simulink model "MX_Gateway"  (Simulink 9.0)
settings: Solver=FixedStepDiscrete  StartTime=0.0  StopTime=100  FixedStep=0.01  RelTol=1e-3
values from neighbouring files (symbols this model uses):
  ACTIVE = Bobcat.Parameter; CreateDefine( ACTIVE                         ,1                          ,'Define' ,'defines.h' )    [defines.m]
  ACTIVE_MODE_ONE = Bobcat.Parameter; CreateDefine( ACTIVE_MODE_ONE                ,19                         ,'Define' ,'defines.h' )    [defines.m]
  AUTHENTICATED = Bobcat.Parameter; CreateDefine( AUTHENTICATED                  ,1                          ,'Define' ,'defines.h' )    [defines.m]
  AUX_EMEA_CONTROLS = Bobcat.Parameter; CreateDefine( AUX_EMEA_CONTROLS  ,1   ,'Define' ,'defines.h' )    [enums.m]
  AUX_FLOW_HIGH = Bobcat.Parameter; CreateDefine( AUX_FLOW_HIGH           ,4  ,'Define' ,'defines.h' )    [enums.m]
  AUX_FLOW_INACTIVE = Bobcat.Parameter; CreateDefine( AUX_FLOW_INACTIVE       ,0  ,'Define' ,'defines.h' )    [enums.m]
  AUX_FLOW_LOW = Bobcat.Parameter; CreateDefine( AUX_FLOW_LOW            ,1  ,'Define' ,'defines.h' )    [enums.m]
  AUX_FLOW_MEDIUM = Bobcat.Parameter; CreateDefine( AUX_FLOW_MEDIUM         ,2  ,'Define' ,'defines.h' )    [enums.m]
  AUX_FLOW_STANDARD = Bobcat.Parameter; CreateDefine( AUX_FLOW_STANDARD       ,3  ,'Define' ,'defines.h' )    [enums.m]
  AUX_NON_FUNCTIONAL = Bobcat.Parameter; CreateDefine( AUX_NON_FUNCTIONAL ,255 ,'Define' ,'defines.h' )    [enums.m]
  BASE_ACTIVE = Bobcat.Parameter; CreateDefine( BASE_ACTIVE                    ,253                        ,'Define' ,'defines.h' )    [defines.m]
  BASE_DETENT = Bobcat.Parameter; CreateDefine( BASE_DETENT                    ,1                          ,'Define' ,'defines.h' )    [defines.m]
  BEEP_NONE = Bobcat.Parameter; CreateDefine( BEEP_NONE                      ,255                        ,'Define' ,'defines.h' )    [defines.m]
  CD_AIR_FILTER_DEBOUNCE_NORMAL = Bobcat.Parameter; CreateAliasCD( CD_AIR_FILTER_DEBOUNCE_NORMAL                        ,uint16(500)                ,'ConstantDataPointer' ,'CONSTANT_DATA' ,'AIR_FILTER_DEBOUNCE_NORMAL')    [cdata.m]    (also in hc12_cdata.m; m4_cdata.m)
  CD_AIR_FILTER_DEBOUNCE_NOT_CONNECTED = Bobcat.Parameter; CreateAliasCD( CD_AIR_FILTER_DEBOUNCE_NOT_CONNECTED                 ,uint16(3000)               ,'ConstantDataPointer' ,'CONSTANT_DATA' ,'AIR_FILTER_DEBOUNCE_NOT_CONNECTED')    [cdata.m]    (also in hc12_cdata.m; m4_cdata.m)
  CD_AIR_FILTER_DEBOUNCE_PLUGGED = Bobcat.Parameter; CreateAliasCD( CD_AIR_FILTER_DEBOUNCE_PLUGGED                       ,uint16(3000)               ,'ConstantDataPointer' ,'CONSTANT_DATA' ,'AIR_FILTER_DEBOUNCE_PLUGGED')    [cdata.m]    (also in hc12_cdata.m; m4_cdata.m)
  CD_AIR_FILTER_FUNCTION = Bobcat.Parameter; CreateAliasCD( CD_AIR_FILTER_FUNCTION                               ,uint8(255)                 ,'ConstantDataPointer' ,'CONSTANT_DATA' ,'AIR_FILTER_FUNCTION')    [cdata.m]    (also in hc12_cdata.m; m4_cdata.m)
  CD_AIR_FILTER_PLUGGED_WEIGHT = Bobcat.Parameter; CreateAliasCD( CD_AIR_FILTER_PLUGGED_WEIGHT                         ,uint8(2)                   ,'ConstantDataPointer' ,'CONSTANT_DATA' ,'AIR_FILTER_PLUGGED_WEIGHT')    [cdata.m]    (also in hc12_cdata.m; m4_cdata.m)
  CD_AIR_FILTER_UNPLUGGED_WEIGHT = Bobcat.Parameter; CreateAliasCD( CD_AIR_FILTER_UNPLUGGED_WEIGHT                       ,uint8(1)                   ,'ConstantDataPointer' ,'CONSTANT_DATA' ,'AIR_FILTER_UNPLUGGED_WEIGHT')    [cdata.m]    (also in hc12_cdata.m; m4_cdata.m)
  CD_AUTOIDLE_ARMBAR_DELAY = Bobcat.Parameter; CreateAliasCD( CD_AUTOIDLE_ARMBAR_DELAY                             ,uint8(2)                   ,'ConstantDataPointer' ,'CONSTANT_DATA' ,'AUTOIDLE_ARMBAR_DELAY')    [cdata.m]    (also in hc12_cdata.m; m4_cdata.m)
  CD_AUTOIDLE_FUNCTION = Bobcat.Parameter; CreateAliasCD( CD_AUTOIDLE_FUNCTION                                 ,uint8(255)                 ,'ConstantDataPointer' ,'CONSTANT_DATA' ,'AUTOIDLE_FUNCTION')    [cdata.m]    (also in hc12_cdata.m; m4_cdata.m)
  CD_AUTOIDLE_IDLE_DELAY = Bobcat.Parameter; CreateAliasCD( CD_AUTOIDLE_IDLE_DELAY                               ,uint8(40)                  ,'ConstantDataPointer' ,'CONSTANT_DATA' ,'AUTOIDLE_IDLE_DELAY')    [cdata.m]    (also in hc12_cdata.m; m4_cdata.m)
  CD_AUTOIDLE_LOW_IDLE_START = Bobcat.Parameter; CreateAliasCD( CD_AUTOIDLE_LOW_IDLE_START                           ,uint8(255)                 ,'ConstantDataPointer' ,'CONSTANT_DATA' ,'AUTOIDLE_LOW_IDLE_START')    [cdata.m]    (also in hc12_cdata.m; m4_cdata.m)
  CD_AUTOIDLE_PRESSURE_OFF = Bobcat.Parameter; CreateAliasCD( CD_AUTOIDLE_PRESSURE_OFF                             ,uint16(300)                ,'ConstantDataPointer' ,'CONSTANT_DATA' ,'AUTOIDLE_PRESSURE_OFF')    [cdata.m]    (also in hc12_cdata.m; m4_cdata.m)
  CD_AUTOIDLE_PRESSURE_ON = Bobcat.Parameter; CreateAliasCD( CD_AUTOIDLE_PRESSURE_ON                              ,uint16(270)                ,'ConstantDataPointer' ,'CONSTANT_DATA' ,'AUTOIDLE_PRESSURE_ON')    [cdata.m]    (also in hc12_cdata.m; m4_cdata.m)
  CD_AUTOSHIFT_FUNCTION = Bobcat.Parameter; CreateAliasCD( CD_AUTOSHIFT_FUNCTION                                ,uint8(255)                 ,'ConstantDataPointer' ,'CONSTANT_DATA' ,'AUTOSHIFT_FUNCTION')    [cdata.m]    (also in hc12_cdata.m; m4_cdata.m)
  CD_AUTOSHIFT_PHIGH1 = Bobcat.Parameter; CreateAliasCD( CD_AUTOSHIFT_PHIGH1                                  ,uint16(205)                ,'ConstantDataPointer' ,'CONSTANT_DATA' ,'AUTOSHIFT_PHIGH1')    [cdata.m]    (also in hc12_cdata.m; m4_cdata.m)
  CD_AUTOSHIFT_PHIGH2 = Bobcat.Parameter; CreateAliasCD( CD_AUTOSHIFT_PHIGH2                                  ,uint16(193)                ,'ConstantDataPointer' ,'CONSTANT_DATA' ,'AUTOSHIFT_PHIGH2')    [cdata.m]    (also in hc12_cdata.m; m4_cdata.m)
  CD_AUTOSHIFT_PHIGH_TEMP1 = Bobcat.Parameter; CreateAliasCD( CD_AUTOSHIFT_PHIGH_TEMP1                             ,int16(70)                  ,'ConstantDataPointer' ,'CONSTANT_DATA' ,'AUTOSHIFT_PHIGH_TEMP1')    [cdata.m]    (also in hc12_cdata.m; m4_cdata.m)
  CD_AUTOSHIFT_PHIGH_TEMP2 = Bobcat.Parameter; CreateAliasCD( CD_AUTOSHIFT_PHIGH_TEMP2                             ,int16(160)                 ,'ConstantDataPointer' ,'CONSTANT_DATA' ,'AUTOSHIFT_PHIGH_TEMP2')    [cdata.m]    (also in hc12_cdata.m; m4_cdata.m)
  CD_AUTOSHIFT_PLOW1 = Bobcat.Parameter; CreateAliasCD( CD_AUTOSHIFT_PLOW1                                   ,uint16(130)                ,'ConstantDataPointer' ,'CONSTANT_DATA' ,'AUTOSHIFT_PLOW1')    [cdata.m]    (also in hc12_cdata.m; m4_cdata.m)
  CD_AUTOSHIFT_PLOW2 = Bobcat.Parameter; CreateAliasCD( CD_AUTOSHIFT_PLOW2                                   ,uint16(100)                ,'ConstantDataPointer' ,'CONSTANT_DATA' ,'AUTOSHIFT_PLOW2')    [cdata.m]    (also in hc12_cdata.m; m4_cdata.m)
  CD_AUTOSHIFT_PLOW_TEMP1 = Bobcat.Parameter; CreateAliasCD( CD_AUTOSHIFT_PLOW_TEMP1                              ,int16(20)                  ,'ConstantDataPointer' ,'CONSTANT_DATA' ,'AUTOSHIFT_PLOW_TEMP1')    [cdata.m]    (also in hc12_cdata.m; m4_cdata.m)
  CD_AUTOSHIFT_PLOW_TEMP2 = Bobcat.Parameter; CreateAliasCD( CD_AUTOSHIFT_PLOW_TEMP2                              ,int16(80)                  ,'ConstantDataPointer' ,'CONSTANT_DATA' ,'AUTOSHIFT_PLOW_TEMP2')    [cdata.m]    (also in hc12_cdata.m; m4_cdata.m)
  CD_AUTOSHIFT_TRAVEL_DELAY = Bobcat.Parameter; CreateAliasCD( CD_AUTOSHIFT_TRAVEL_DELAY                            ,uint8(32)                  ,'ConstantDataPointer' ,'CONSTANT_DATA' ,'AUTOSHIFT_TRAVEL_DELAY')    [cdata.m]    (also in hc12_cdata.m; m4_cdata.m)
  CD_AUX2_CRACKPOINT_CURRENT = Bobcat.Parameter; CreateAliasCD( CD_AUX2_CRACKPOINT_CURRENT                           ,fi(0,0,16,12,'hex','0c28') ,'ConstantDataPointer' ,'CONSTANT_DATA' ,'AUX2_CRACKPOINT_CURRENT')    [cdata.m]    (also in hc12_cdata.m; m4_cdata.m)
  CD_AUX2_FLOW_LOW_FUNCTION = Bobcat.Parameter; CreateAliasCD( CD_AUX2_FLOW_LOW_FUNCTION                            ,uint8(255)                 ,'ConstantDataPointer' ,'CONSTANT_DATA' ,'AUX2_FLOW_LOW_FUNCTION')    [cdata.m]    (also in hc12_cdata.m; m4_cdata.m)
  CD_AUX2_FLOW_MED_FUNCTION = Bobcat.Parameter; CreateAliasCD( CD_AUX2_FLOW_MED_FUNCTION                            ,uint8(255)                 ,'ConstantDataPointer' ,'CONSTANT_DATA' ,'AUX2_FLOW_MED_FUNCTION')    [cdata.m]    (also in hc12_cdata.m; m4_cdata.m)
  CD_AUX2_HI_FLOW_PERCENT = Bobcat.Parameter; CreateAliasCD( CD_AUX2_HI_FLOW_PERCENT                              ,fi(0,0,16,16,'hex','ffff') ,'ConstantDataPointer' ,'CONSTANT_DATA' ,'AUX2_HI_FLOW_PERCENT')    [cdata.m]    (also in hc12_cdata.m; m4_cdata.m)
  CD_AUX2_LOW_FLOW_PERCENT = Bobcat.Parameter; CreateAliasCD( CD_AUX2_LOW_FLOW_PERCENT                             ,fi(0,0,16,16,'hex','7333') ,'ConstantDataPointer' ,'CONSTANT_DATA' ,'AUX2_LOW_FLOW_PERCENT')    [cdata.m]    (also in hc12_cdata.m; m4_cdata.m)
  CD_AUX2_MAX_FLOW_CURRENT = Bobcat.Parameter; CreateAliasCD( CD_AUX2_MAX_FLOW_CURRENT                             ,fi(0,0,16,12,'hex','14cc') ,'ConstantDataPointer' ,'CONSTANT_DATA' ,'AUX2_MAX_FLOW_CURRENT')    [cdata.m]    (also in hc12_cdata.m; m4_cdata.m)
  CD_AUX2_MED_FLOW_PERCENT = Bobcat.Parameter; CreateAliasCD( CD_AUX2_MED_FLOW_PERCENT                             ,fi(0,0,16,16,'hex','9999') ,'ConstantDataPointer' ,'CONSTANT_DATA' ,'AUX2_MED_FLOW_PERCENT')    [cdata.m]    (also in hc12_cdata.m; m4_cdata.m)
  CD_AUX_CRACKPOINT_CURRENT = Bobcat.Parameter; CreateAliasCD( CD_AUX_CRACKPOINT_CURRENT                            ,fi(0,0,16,12,'hex','0000') ,'ConstantDataPointer' ,'CONSTANT_DATA' ,'AUX_CRACKPOINT_CURRENT')    [cdata.m]    (also in hc12_cdata.m; m4_cdata.m)
  CD_AUX_DETENT_FLASH_TIME = Bobcat.Parameter; CreateAliasCD( CD_AUX_DETENT_FLASH_TIME                             ,uint16(72)                 ,'ConstantDataPointer' ,'CONSTANT_DATA' ,'AUX_DETENT_FLASH_TIME')    [cdata.m]    (also in hc12_cdata.m; m4_cdata.m)
  CD_AUX_DETENT_HOLD_TIME = Bobcat.Parameter; CreateAliasCD( CD_AUX_DETENT_HOLD_TIME                              ,uint16(100)                ,'ConstantDataPointer' ,'CONSTANT_DATA' ,'AUX_DETENT_HOLD_TIME')    [cdata.m]    (also in hc12_cdata.m; m4_cdata.m)
  CD_AUX_FULL_ON_AUX1 = Bobcat.Parameter; CreateAliasCD( CD_AUX_FULL_ON_AUX1                                  ,fi(0,0,16,12,'hex','10cd') ,'ConstantDataPointer' ,'CONSTANT_DATA' ,'AUX_FULL_ON_AUX1')    [cdata.m]    (also in hc12_cdata.m; m4_cdata.m)
  CD_AUX_FULL_ON_AUX2 = Bobcat.Parameter; CreateAliasCD( CD_AUX_FULL_ON_AUX2                                  ,fi(0,0,16,12,'hex','139d') ,'ConstantDataPointer' ,'CONSTANT_DATA' ,'AUX_FULL_ON_AUX2')    [cdata.m]    (also in hc12_cdata.m; m4_cdata.m)
  CD_AUX_FULL_ON_AUX3 = Bobcat.Parameter; CreateAliasCD( CD_AUX_FULL_ON_AUX3                                  ,fi(0,0,16,12,'hex','17d7') ,'ConstantDataPointer' ,'CONSTANT_DATA' ,'AUX_FULL_ON_AUX3')    [cdata.m]    (also in hc12_cdata.m; m4_cdata.m)
  CD_AUX_FUNCTION = Bobcat.Parameter; CreateAliasCD( CD_AUX_FUNCTION                                      ,uint8(0)                   ,'ConstantDataPointer' ,'CONSTANT_DATA' ,'AUX_FUNCTION')    [cdata.m]    (also in hc12_cdata.m; m4_cdata.m)
  CD_AUX_RATE_LIMIT_CRACKPOINT = Bobcat.Parameter; CreateAliasCD( CD_AUX_RATE_LIMIT_CRACKPOINT                         ,fi(0,0,16,12,'hex','0800') ,'ConstantDataPointer' ,'CONSTANT_DATA' ,'AUX_RATE_LIMIT_CRACKPOINT')    [cdata.m]    (also in hc12_cdata.m; m4_cdata.m)
  CD_AUX_RATE_LIMIT_CRACKPOINT_RATE = Bobcat.Parameter; CreateAliasCD( CD_AUX_RATE_LIMIT_CRACKPOINT_RATE                    ,fi(0,1,32,12,'int',102400) ,'ConstantDataPointer' ,'CONSTANT_DATA' ,'AUX_RATE_LIMIT_CRACKPOINT_RATE')    [cdata.m]    (also in hc12_cdata.m; m4_cdata.m)
  CD_AUX_RATE_LIMIT_FUNCTION = Bobcat.Parameter; CreateAliasCD( CD_AUX_RATE_LIMIT_FUNCTION                           ,uint8(255)                 ,'ConstantDataPointer' ,'CONSTANT_DATA' ,'AUX_RATE_LIMIT_FUNCTION')    [cdata.m]    (also in hc12_cdata.m; m4_cdata.m)
  CD_AUX_RATE_LIMIT_MAX_ENG_SPD = Bobcat.Parameter; CreateAliasCD( CD_AUX_RATE_LIMIT_MAX_ENG_SPD                        ,uint16(1600)               ,'ConstantDataPointer' ,'CONSTANT_DATA' ,'AUX_RATE_LIMIT_MAX_ENG_SPD')    [cdata.m]    (also in hc12_cdata.m; m4_cdata.m)
  CD_AUX_RATE_LIMIT_MIN_ENG_SPD = Bobcat.Parameter; CreateAliasCD( CD_AUX_RATE_LIMIT_MIN_ENG_SPD                        ,uint16(100)                ,'ConstantDataPointer' ,'CONSTANT_DATA' ,'AUX_RATE_LIMIT_MIN_ENG_SPD')    [cdata.m]    (also in hc12_cdata.m; m4_cdata.m)
  CD_AUX_RATE_LIMIT_NORMAL_RATE = Bobcat.Parameter; CreateAliasCD( CD_AUX_RATE_LIMIT_NORMAL_RATE                        ,fi(0,1,32,12,'int',2048)   ,'ConstantDataPointer' ,'CONSTANT_DATA' ,'AUX_RATE_LIMIT_NORMAL_RATE')    [cdata.m]    (also in hc12_cdata.m; m4_cdata.m)
  CD_AUXPOT_CAL_DEADBAND_HIGH = Bobcat.Parameter; CreateAliasCD( CD_AUXPOT_CAL_DEADBAND_HIGH                          ,uint16(574)                ,'ConstantDataPointer' ,'CONSTANT_DATA' ,'AUXPOT_CAL_DEADBAND_HIGH')    [cdata.m]    (also in hc12_cdata.m; m4_cdata.m)
  CD_AUXPOT_CAL_DEADBAND_LOW = Bobcat.Parameter; CreateAliasCD( CD_AUXPOT_CAL_DEADBAND_LOW                           ,uint16(424)                ,'ConstantDataPointer' ,'CONSTANT_DATA' ,'AUXPOT_CAL_DEADBAND_LOW')    [cdata.m]    (also in hc12_cdata.m; m4_cdata.m)
  CD_AUXPOT_CENTER_RATIO = Bobcat.Parameter; CreateAliasCD( CD_AUXPOT_CENTER_RATIO                               ,uint16(500)                ,'ConstantDataPointer' ,'CONSTANT_DATA' ,'AUXPOT_CENTER_RATIO')    [cdata.m]    (also in hc12_cdata.m; m4_cdata.m)
  CD_AUXPOT_DEADBAND = Bobcat.Parameter; CreateAliasCD( CD_AUXPOT_DEADBAND                                   ,uint8(38)                  ,'ConstantDataPointer' ,'CONSTANT_DATA' ,'AUXPOT_DEADBAND')    [cdata.m]    (also in hc12_cdata.m; m4_cdata.m)
  CD_AUXPOT_FUNCTION = Bobcat.Parameter; CreateAliasCD( CD_AUXPOT_FUNCTION                                   ,uint8(0)                   ,'ConstantDataPointer' ,'CONSTANT_DATA' ,'AUXPOT_FUNCTION')    [cdata.m]    (also in hc12_cdata.m; m4_cdata.m)
  CD_AUXPOT_RATIO_GAIN = Bobcat.Parameter; CreateAliasCD( CD_AUXPOT_RATIO_GAIN                                 ,uint16(1000)               ,'ConstantDataPointer' ,'CONSTANT_DATA' ,'AUXPOT_RATIO_GAIN')    [cdata.m]    (also in hc12_cdata.m; m4_cdata.m)
  CD_CAN_THROTTLE_FUNCTION = Bobcat.Parameter; CreateAliasCD( CD_CAN_THROTTLE_FUNCTION                             ,uint8(255)                 ,'ConstantDataPointer' ,'CONSTANT_DATA' ,'CAN_THROTTLE_FUNCTION')    [cdata.m]    (also in hc12_cdata.m; m4_cdata.m)
  CD_CAN_THROTTLE_REVERSE = Bobcat.Parameter; CreateAliasCD( CD_CAN_THROTTLE_REVERSE                              ,uint8(255)                 ,'ConstantDataPointer' ,'CONSTANT_DATA' ,'CAN_THROTTLE_REVERSE')    [cdata.m]    (also in hc12_cdata.m; m4_cdata.m)
  CD_CONSOLE_DEBOUNCE_ABSENT = Bobcat.Parameter; CreateAliasCD( CD_CONSOLE_DEBOUNCE_ABSENT                           ,uint16(12)                 ,'ConstantDataPointer' ,'CONSTANT_DATA' ,'CONSOLE_DEBOUNCE_ABSENT')    [cdata.m]    (also in hc12_cdata.m; m4_cdata.m)
  CD_CONSOLE_DEBOUNCE_OORH = Bobcat.Parameter; CreateAliasCD( CD_CONSOLE_DEBOUNCE_OORH                             ,uint16(12)                 ,'ConstantDataPointer' ,'CONSTANT_DATA' ,'CONSOLE_DEBOUNCE_OORH')    [cdata.m]    (also in hc12_cdata.m; m4_cdata.m)
  CD_CONSOLE_DEBOUNCE_OORL = Bobcat.Parameter; CreateAliasCD( CD_CONSOLE_DEBOUNCE_OORL                             ,uint16(12)                 ,'ConstantDataPointer' ,'CONSTANT_DATA' ,'CONSOLE_DEBOUNCE_OORL')    [cdata.m]    (also in hc12_cdata.m; m4_cdata.m)
  CD_CONSOLE_DEBOUNCE_PRESENT = Bobcat.Parameter; CreateAliasCD( CD_CONSOLE_DEBOUNCE_PRESENT                          ,uint16(12)                 ,'ConstantDataPointer' ,'CONSTANT_DATA' ,'CONSOLE_DEBOUNCE_PRESENT')    [cdata.m]    (also in hc12_cdata.m; m4_cdata.m)
  CD_CONSOLE_FUNCTION = Bobcat.Parameter; CreateAliasCD( CD_CONSOLE_FUNCTION                                  ,uint8(0)                   ,'ConstantDataPointer' ,'CONSTANT_DATA' ,'CONSOLE_FUNCTION')    [cdata.m]    (also in hc12_cdata.m; m4_cdata.m)
  CD_CONSOLE_OORH = Bobcat.Parameter; CreateAliasCD( CD_CONSOLE_OORH                                      ,uint16(740)                ,'ConstantDataPointer' ,'CONSTANT_DATA' ,'CONSOLE_OORH')    [cdata.m]    (also in hc12_cdata.m; m4_cdata.m)
  CD_CONSOLE_OORL = Bobcat.Parameter; CreateAliasCD( CD_CONSOLE_OORL                                      ,uint16(10)                 ,'ConstantDataPointer' ,'CONSTANT_DATA' ,'CONSOLE_OORL')    [cdata.m]    (also in hc12_cdata.m; m4_cdata.m)
  CD_CONSOLE_PRESENT_HIGH = Bobcat.Parameter; CreateAliasCD( CD_CONSOLE_PRESENT_HIGH                              ,uint16(515)                ,'ConstantDataPointer' ,'CONSTANT_DATA' ,'CONSOLE_PRESENT_HIGH')    [cdata.m]    (also in hc12_cdata.m; m4_cdata.m)
  CD_CONSOLE_PRESENT_LOW = Bobcat.Parameter; CreateAliasCD( CD_CONSOLE_PRESENT_LOW                               ,uint16(375)                ,'ConstantDataPointer' ,'CONSTANT_DATA' ,'CONSOLE_PRESENT_LOW')    [cdata.m]    (also in hc12_cdata.m; m4_cdata.m)
  CD_DELUXE_GEN5_5_FUNCTION = Bobcat.Parameter; CreateAliasCD( CD_DELUXE_GEN5_5_FUNCTION                            ,uint8(255)                 ,'ConstantDataPointer' ,'CONSTANT_DATA' ,'DELUXE_GEN5_5_FUNCTION')    [cdata.m]    (also in hc12_cdata.m; m4_cdata.m)
  CD_DELUXE_GEN5_FUNCTION = Bobcat.Parameter; CreateAliasCD( CD_DELUXE_GEN5_FUNCTION                              ,uint8(255)                 ,'ConstantDataPointer' ,'CONSTANT_DATA' ,'DELUXE_GEN5_FUNCTION')    [cdata.m]    (also in hc12_cdata.m; m4_cdata.m)
  CD_DERATE_ENGINE_SPEED_DEBOUNCE_HIGH = Bobcat.Parameter; CreateAliasCD( CD_DERATE_ENGINE_SPEED_DEBOUNCE_HIGH                 ,uint16(200)                ,'ConstantDataPointer' ,'CONSTANT_DATA' ,'DERATE_ENGINE_SPEED_DEBOUNCE_HIGH')    [cdata.m]    (also in hc12_cdata.m; m4_cdata.m)
  CD_DERATE_ENGINE_SPEED_DEBOUNCE_SHUTDOWN = Bobcat.Parameter; CreateAliasCD( CD_DERATE_ENGINE_SPEED_DEBOUNCE_SHUTDOWN             ,uint16(1000)               ,'ConstantDataPointer' ,'CONSTANT_DATA' ,'DERATE_ENGINE_SPEED_DEBOUNCE_SHUTDOWN')    [cdata.m]    (also in hc12_cdata.m; m4_cdata.m)
  CD_DERATE_ENGINE_SPEED_HIGH_OFFSET = Bobcat.Parameter; CreateAliasCD( CD_DERATE_ENGINE_SPEED_HIGH_OFFSET                   ,uint16(200)                ,'ConstantDataPointer' ,'CONSTANT_DATA' ,'DERATE_ENGINE_SPEED_HIGH_OFFSET')    [cdata.m]    (also in hc12_cdata.m; m4_cdata.m)
  CD_DERATE_METHOD = Bobcat.Parameter; CreateAliasCD( CD_DERATE_METHOD                                     ,uint8(0)                   ,'ConstantDataPointer' ,'CONSTANT_DATA' ,'DERATE_METHOD')    [cdata.m]    (also in hc12_cdata.m; m4_cdata.m)
  CD_DUMP_VALVE_FUNCTION = Bobcat.Parameter; CreateAliasCD( CD_DUMP_VALVE_FUNCTION                               ,uint8(255)                 ,'ConstantDataPointer' ,'CONSTANT_DATA' ,'DUMP_VALVE_FUNCTION')    [cdata.m]    (also in hc12_cdata.m; m4_cdata.m)
  CD_DUMP_VALVE_ON_CURRENT = Bobcat.Parameter; CreateAliasCD( CD_DUMP_VALVE_ON_CURRENT                             ,fi(0,0,16,12,'hex','ffff') ,'ConstantDataPointer' ,'CONSTANT_DATA' ,'DUMP_VALVE_ON_CURRENT')    [cdata.m]    (also in hc12_cdata.m; m4_cdata.m)
  CD_ECO_MODE_THROTTLE_REDUCTION = Bobcat.Parameter; CreateAliasCD( CD_ECO_MODE_THROTTLE_REDUCTION                       ,uint16(850)                ,'ConstantDataPointer' ,'CONSTANT_DATA' ,'ECO_MODE_THROTTLE_REDUCTION')    [cdata.m]    (also in hc12_cdata.m; m4_cdata.m)
  CD_ECT_FILTER_GAIN = Bobcat.Parameter; CreateAliasCD( CD_ECT_FILTER_GAIN                                   ,uint8(6)                   ,'ConstantDataPointer' ,'CONSTANT_DATA' ,'ECT_FILTER_GAIN')    [cdata.m]    (also in hc12_cdata.m; m4_cdata.m)
  CD_ECU_FUNCTION = Bobcat.Parameter; CreateAliasCD( CD_ECU_FUNCTION                                      ,uint8(255)                 ,'ConstantDataPointer' ,'CONSTANT_DATA' ,'ECU_FUNCTION')    [cdata.m]    (also in hc12_cdata.m; m4_cdata.m)
  CD_ECU_HOLD_DEBOUNCE_ERROR_ON = Bobcat.Parameter; CreateAliasCD( CD_ECU_HOLD_DEBOUNCE_ERROR_ON                        ,uint16(20)                 ,'ConstantDataPointer' ,'CONSTANT_DATA' ,'ECU_HOLD_DEBOUNCE_ERROR_ON')    [cdata.m]    (also in hc12_cdata.m; m4_cdata.m)
  CD_EMEA_CONTROLS_HOLD_TIME = Bobcat.Parameter; CreateAliasCD( CD_EMEA_CONTROLS_HOLD_TIME                           ,uint16(30)                 ,'ConstantDataPointer' ,'CONSTANT_DATA' ,'EMEA_CONTROLS_HOLD_TIME')    [cdata.m]    (also in hc12_cdata.m; m4_cdata.m)
  CD_EMEA_CONTROLS_OPTION_A = Bobcat.Parameter; CreateAliasCD( CD_EMEA_CONTROLS_OPTION_A                            ,uint8(255)                 ,'ConstantDataPointer' ,'CONSTANT_DATA' ,'EMEA_CONTROLS_OPTION_A')    [cdata.m]    (also in hc12_cdata.m; m4_cdata.m)
  CD_EMEA_CONTROLS_OPTION_C = Bobcat.Parameter; CreateAliasCD( CD_EMEA_CONTROLS_OPTION_C                            ,uint8(255)                 ,'ConstantDataPointer' ,'CONSTANT_DATA' ,'EMEA_CONTROLS_OPTION_C')    [cdata.m]    (also in hc12_cdata.m; m4_cdata.m)
  CD_EMEA_CONTROLS_REVERSE = Bobcat.Parameter; CreateAliasCD( CD_EMEA_CONTROLS_REVERSE                             ,uint8(255)                 ,'ConstantDataPointer' ,'CONSTANT_DATA' ,'EMEA_CONTROLS_REVERSE')    [cdata.m]    (also in hc12_cdata.m; m4_cdata.m)
  CD_EMEA_ELECTRIC_VERSION = Bobcat.Parameter; CreateAliasCD( CD_EMEA_ELECTRIC_VERSION                             ,uint8(0)                   ,'ConstantDataPointer' ,'CONSTANT_DATA' ,'EMEA_ELECTRIC_VERSION')    [cdata.m]    (also in hc12_cdata.m; m4_cdata.m)
  CD_EMEA_POT_CAL_DEADBAND_HIGH = Bobcat.Parameter; CreateAliasCD( CD_EMEA_POT_CAL_DEADBAND_HIGH                        ,uint16(574)                ,'ConstantDataPointer' ,'CONSTANT_DATA' ,'EMEA_POT_CAL_DEADBAND_HIGH')    [cdata.m]    (also in hc12_cdata.m; m4_cdata.m)
  CD_EMEA_POT_CAL_DEADBAND_LOW = Bobcat.Parameter; CreateAliasCD( CD_EMEA_POT_CAL_DEADBAND_LOW                         ,uint16(424)                ,'ConstantDataPointer' ,'CONSTANT_DATA' ,'EMEA_POT_CAL_DEADBAND_LOW')    [cdata.m]    (also in hc12_cdata.m; m4_cdata.m)
  CD_ENGINE_RPM_FACTOR = Bobcat.Parameter; CreateAliasCD( CD_ENGINE_RPM_FACTOR                                 ,uint8(35)                  ,'ConstantDataPointer' ,'CONSTANT_DATA' ,'ENGINE_RPM_FACTOR')    [cdata.m]    (also in hc12_cdata.m; m4_cdata.m)
  CD_ENGINE_SPEED_FILTER_FAST_VALUE = Bobcat.Parameter; CreateAliasCD( CD_ENGINE_SPEED_FILTER_FAST_VALUE                    ,uint8(1)                   ,'ConstantDataPointer' ,'CONSTANT_DATA' ,'ENGINE_SPEED_FILTER_FAST_VALUE')    [cdata.m]    (also in hc12_cdata.m; m4_cdata.m)
  CD_ENGINE_SPEED_FILTER_SLOW_VALUE = Bobcat.Parameter; CreateAliasCD( CD_ENGINE_SPEED_FILTER_SLOW_VALUE                    ,uint8(4)                   ,'ConstantDataPointer' ,'CONSTANT_DATA' ,'ENGINE_SPEED_FILTER_SLOW_VALUE')    [cdata.m]    (also in hc12_cdata.m; m4_cdata.m)
  CD_ENGINE_SPEED_RUNNING = Bobcat.Parameter; CreateAliasCD( CD_ENGINE_SPEED_RUNNING                              ,uint16(800)                ,'ConstantDataPointer' ,'CONSTANT_DATA' ,'ENGINE_SPEED_RUNNING')    [cdata.m]    (also in hc12_cdata.m; m4_cdata.m)
  CD_ENGINE_START_DELAY_DEBOUNCE_RPM_NO_SIGNAL = Bobcat.Parameter; CreateAliasCD( CD_ENGINE_START_DELAY_DEBOUNCE_RPM_NO_SIGNAL         ,uint16(0)                  ,'ConstantDataPointer' ,'CONSTANT_DATA' ,'ENGINE_START_DELAY_DEBOUNCE_RPM_NO_SIGNAL')    [cdata.m]    (also in hc12_cdata.m; m4_cdata.m)
  CD_ENGINE_START_DELAY_OFF_TIME = Bobcat.Parameter; CreateAliasCD( CD_ENGINE_START_DELAY_OFF_TIME                       ,uint16(0)                  ,'ConstantDataPointer' ,'CONSTANT_DATA' ,'ENGINE_START_DELAY_OFF_TIME')    [cdata.m]    (also in hc12_cdata.m; m4_cdata.m)
  CD_ENGINE_START_DELAY_RPM_NO_SIGNAL = Bobcat.Parameter; CreateAliasCD( CD_ENGINE_START_DELAY_RPM_NO_SIGNAL                  ,uint16(0)                  ,'ConstantDataPointer' ,'CONSTANT_DATA' ,'ENGINE_START_DELAY_RPM_NO_SIGNAL')    [cdata.m]    (also in hc12_cdata.m; m4_cdata.m)
  CD_EOP_DEBOUNCE_EXTREMELY_LOW = Bobcat.Parameter; CreateAliasCD( CD_EOP_DEBOUNCE_EXTREMELY_LOW                        ,uint16(50)                 ,'ConstantDataPointer' ,'CONSTANT_DATA' ,'EOP_DEBOUNCE_EXTREMELY_LOW')    [cdata.m]    (also in hc12_cdata.m; m4_cdata.m)
  CD_EOP_DEBOUNCE_NORMAL = Bobcat.Parameter; CreateAliasCD( CD_EOP_DEBOUNCE_NORMAL                               ,uint16(50)                 ,'ConstantDataPointer' ,'CONSTANT_DATA' ,'EOP_DEBOUNCE_NORMAL')    [cdata.m]    (also in hc12_cdata.m; m4_cdata.m)
  CD_EOP_DEBOUNCE_SHUTDOWN = Bobcat.Parameter; CreateAliasCD( CD_EOP_DEBOUNCE_SHUTDOWN                             ,uint16(100)                ,'ConstantDataPointer' ,'CONSTANT_DATA' ,'EOP_DEBOUNCE_SHUTDOWN')    [cdata.m]    (also in hc12_cdata.m; m4_cdata.m)
  CD_EOP_FILTER_VALUE = Bobcat.Parameter; CreateAliasCD( CD_EOP_FILTER_VALUE                                  ,uint8(3)                   ,'ConstantDataPointer' ,'CONSTANT_DATA' ,'EOP_FILTER_VALUE')    [cdata.m]    (also in hc12_cdata.m; m4_cdata.m)
  CD_EOP_FUNCTION = Bobcat.Parameter; CreateAliasCD( CD_EOP_FUNCTION                                      ,uint8(1)                   ,'ConstantDataPointer' ,'CONSTANT_DATA' ,'EOP_FUNCTION')    [cdata.m]    (also in hc12_cdata.m; m4_cdata.m)
  CD_EOP_SWITCH_GAUGE_VALUE = Bobcat.Parameter; CreateAliasCD( CD_EOP_SWITCH_GAUGE_VALUE                            ,uint16(125)                ,'ConstantDataPointer' ,'CONSTANT_DATA' ,'EOP_SWITCH_GAUGE_VALUE')    [cdata.m]    (also in hc12_cdata.m; m4_cdata.m)
  CD_EOP_SWITCH_LOW = Bobcat.Parameter; CreateAliasCD( CD_EOP_SWITCH_LOW                                    ,uint16(50)                 ,'ConstantDataPointer' ,'CONSTANT_DATA' ,'EOP_SWITCH_LOW')    [cdata.m]    (also in hc12_cdata.m; m4_cdata.m)
  CD_EOP_SWITCH_PRESSURE_VALUE = Bobcat.Parameter; CreateAliasCD( CD_EOP_SWITCH_PRESSURE_VALUE                         ,uint16(40)                 ,'ConstantDataPointer' ,'CONSTANT_DATA' ,'EOP_SWITCH_PRESSURE_VALUE')    [cdata.m]    (also in hc12_cdata.m; m4_cdata.m)
  CD_EOT_DEBOUNCE_EXTREMELY_LOW = Bobcat.Parameter; CreateAliasCD( CD_EOT_DEBOUNCE_EXTREMELY_LOW                        ,uint16(2)                  ,'ConstantDataPointer' ,'CONSTANT_DATA' ,'EOT_DEBOUNCE_EXTREMELY_LOW')    [cdata.m]    (also in hc12_cdata.m; m4_cdata.m)
  CD_EOT_DEBOUNCE_LOW = Bobcat.Parameter; CreateAliasCD( CD_EOT_DEBOUNCE_LOW                                  ,uint16(2)                  ,'ConstantDataPointer' ,'CONSTANT_DATA' ,'EOT_DEBOUNCE_LOW')    [cdata.m]    (also in hc12_cdata.m; m4_cdata.m)
  CD_EOT_DEBOUNCE_NORMAL = Bobcat.Parameter; CreateAliasCD( CD_EOT_DEBOUNCE_NORMAL                               ,uint16(2)                  ,'ConstantDataPointer' ,'CONSTANT_DATA' ,'EOT_DEBOUNCE_NORMAL')    [cdata.m]    (also in hc12_cdata.m; m4_cdata.m)
  CD_EOT_EXTREMELY_LOW = Bobcat.Parameter; CreateAliasCD( CD_EOT_EXTREMELY_LOW                                 ,uint16(81)                 ,'ConstantDataPointer' ,'CONSTANT_DATA' ,'EOT_EXTREMELY_LOW')    [cdata.m]    (also in hc12_cdata.m; m4_cdata.m)
  CD_EOT_FUNCTION = Bobcat.Parameter; CreateAliasCD( CD_EOT_FUNCTION                                      ,uint8(255)                 ,'ConstantDataPointer' ,'CONSTANT_DATA' ,'EOT_FUNCTION')    [cdata.m]    (also in hc12_cdata.m; m4_cdata.m)
  CD_EOT_LOW = Bobcat.Parameter; CreateAliasCD( CD_EOT_LOW                                           ,uint16(90)                 ,'ConstantDataPointer' ,'CONSTANT_DATA' ,'EOT_LOW')    [cdata.m]    (also in hc12_cdata.m; m4_cdata.m)
  CD_EOT_TEMPERATURE_HYSTERESIS = Bobcat.Parameter; CreateAliasCD( CD_EOT_TEMPERATURE_HYSTERESIS                        ,uint16(2)                  ,'ConstantDataPointer' ,'CONSTANT_DATA' ,'EOT_TEMPERATURE_HYSTERESIS')    [cdata.m]    (also in hc12_cdata.m; m4_cdata.m)
  CD_FAST_TRAVEL_FUNCTION = Bobcat.Parameter; CreateAliasCD( CD_FAST_TRAVEL_FUNCTION                              ,uint8(255)                 ,'ConstantDataPointer' ,'CONSTANT_DATA' ,'FAST_TRAVEL_FUNCTION')    [cdata.m]    (also in hc12_cdata.m; m4_cdata.m)
  CD_FAST_TRAVEL_THROTTLE_DELAY = Bobcat.Parameter; CreateAliasCD( CD_FAST_TRAVEL_THROTTLE_DELAY                        ,uint16(10)                 ,'ConstantDataPointer' ,'CONSTANT_DATA' ,'FAST_TRAVEL_THROTTLE_DELAY')    [cdata.m]    (also in hc12_cdata.m; m4_cdata.m)
  CD_FAST_TRAVEL_THROTTLE_LIMIT = Bobcat.Parameter; CreateAliasCD( CD_FAST_TRAVEL_THROTTLE_LIMIT                        ,uint16(1000)               ,'ConstantDataPointer' ,'CONSTANT_DATA' ,'FAST_TRAVEL_THROTTLE_LIMIT')    [cdata.m]    (also in hc12_cdata.m; m4_cdata.m)
  CD_FLYWHEEL_TEETH = Bobcat.Parameter; CreateAliasCD( CD_FLYWHEEL_TEETH                                    ,uint8(83)                  ,'ConstantDataPointer' ,'CONSTANT_DATA' ,'FLYWHEEL_TEETH')    [cdata.m]    (also in hc12_cdata.m; m4_cdata.m)
  CD_FUEL_LEVEL_FILTER_FAST_VALUE = Bobcat.Parameter; CreateAliasCD( CD_FUEL_LEVEL_FILTER_FAST_VALUE                      ,uint8(5)                   ,'ConstantDataPointer' ,'CONSTANT_DATA' ,'FUEL_LEVEL_FILTER_FAST_VALUE')    [cdata.m]    (also in hc12_cdata.m; m4_cdata.m)
  CD_FUEL_LEVEL_FILTER_SLOW_VALUE = Bobcat.Parameter; CreateAliasCD( CD_FUEL_LEVEL_FILTER_SLOW_VALUE                      ,uint8(10)                  ,'ConstantDataPointer' ,'CONSTANT_DATA' ,'FUEL_LEVEL_FILTER_SLOW_VALUE')    [cdata.m]    (also in hc12_cdata.m; m4_cdata.m)
  CD_FUEL_PRIMARY_FEEDBACK_FUNCTION = Bobcat.Parameter; CreateAliasCD( CD_FUEL_PRIMARY_FEEDBACK_FUNCTION                    ,uint8(0)                   ,'ConstantDataPointer' ,'CONSTANT_DATA' ,'FUEL_PRIMARY_FEEDBACK_FUNCTION')    [cdata.m]    (also in hc12_cdata.m; m4_cdata.m)
  ... (+246 more)
undefined symbols (not defined in the file or in its folder): sigbuilder_block
undefined symbols (not defined in the file): CD_FUEL_RESOLUTION_TIMER
libraries: simulink x186, MX_Library x107
(part 37 of 48)

== stateflow chart MX_Gtwy_Control/System/Keyless_Start/Panel_Lock ==
  state KEYPAD [parallel]
    settings: executionOrder=1
    state NOT_PRESSED
    state PRESSED
      state BUTTON_9
        en:pwd_Keyless[pwd_Position] = ASCII_9;
        en:pwd_Position++;
      state BUTTON_8
        en:pwd_Keyless[pwd_Position] = ASCII_8;
        en:pwd_Position++;
      state BUTTON_74
        en:pwd_Keyless[pwd_Position] = ASCII_7;
        en:pwd_Position++;
      state BUTTON_0
        en:pwd_Keyless[pwd_Position] = ASCII_0;
        en:pwd_Position++;
      state NONE
      state BUTTON_1
        en:pwd_Keyless[pwd_Position] = ASCII_1;
        en:pwd_Position++;
      state BUTTON_6
        en:pwd_Keyless[pwd_Position] = ASCII_6;
        en:pwd_Position++;
      state BUTTON_5
        en:pwd_Keyless[pwd_Position] = ASCII_5;
        en:pwd_Position++;
      state BUTTON_2
        en:pwd_Keyless[pwd_Position] = ASCII_2;
        en:pwd_Position++;
      state BUTTON_4
        en:pwd_Keyless[pwd_Position] = ASCII_4;
        en:pwd_Position++;
      state BUTTON_3
        en:pwd_Keyless[pwd_Position] = ASCII_3;
        en:pwd_Position++;
      NONE -> BUTTON_9 [order 1] : [((can_Keypad[2] & KEYPAD_MASK_9) == KEYPAD_PRESSED_9)]
      NONE -> BUTTON_8 [order 2] : [((can_Keypad[1] & KEYPAD_MASK_8) == KEYPAD_PRESSED_8)]
      NONE -> BUTTON_74 [order 3] : [((can_Keypad[1] & KEYPAD_MASK_7) == KEYPAD_PRESSED_7)]
      NONE -> BUTTON_0 [order 10] : [((can_Keypad[2] & KEYPAD_MASK_0) == KEYPAD_PRESSED_0)]
      (default) -> NONE
      NONE -> BUTTON_6 [order 4] : [((can_Keypad[1] & KEYPAD_MASK_6) == KEYPAD_PRESSED_6)]
      NONE -> BUTTON_1 [order 9] : [((can_Keypad[0] & KEYPAD_MASK_1) == KEYPAD_PRESSED_1)]
      NONE -> BUTTON_2 [order 8] : [((can_Keypad[0] & KEYPAD_MASK_2) == KEYPAD_PRESSED_2)]
      NONE -> BUTTON_5 [order 5] : [((can_Keypad[1] & KEYPAD_MASK_5) == KEYPAD_PRESSED_5)]
      NONE -> BUTTON_3 [order 7] : [((can_Keypad[0] & KEYPAD_MASK_3) == KEYPAD_PRESSED_3)]
      NONE -> BUTTON_4 [order 6] : [((can_Keypad[0] & KEYPAD_MASK_4) == KEYPAD_PRESSED_4)]
    state RESET
      en:pwd_Position = 0;
      en:pwd_Keyless = 0;
      en:reset_PwdState = 1;
    (default) -> NOT_PRESSED
    NOT_PRESSED -> PRESSED [order 1] : [((can_Keypad[0] != 0) || (can_Keypad[1] != 0) || (can_Keypad[2] != 0))...
        | && ((state_KSPassword == ENTER_PWD) || (state_KSPassword == ENTER_OWNER1) || (state_KSPassword == ENTER_OWNER2))]
    PRESSED -> NOT_PRESSED : [(can_Keypad[0] == 0)...
        | && (can_Keypad[1] == 0)...
        | && (can_Keypad[2] == 0)]
    RESET -> NOT_PRESSED : after(200,tick)[(state_KSPassword != FAIL)]
    NOT_PRESSED -> RESET [order 2] : [(state_KSPassword == FAIL)]
  state KS_PASSWORD [parallel]
    settings: executionOrder=2
    state CHANGE
      en:state_KSPassword = ENTER_OWNER1;
      state WAIT_NEW1
        en:state_KSPassword = FAIL;
      state ENTER_OWNER
        en:KSChangeDisp = DISPLAY_CODE;
        state DISPLAY_CODE
          en:KSChangeDisp = DISPLAY_CODE;
        state DISPLAY_ASTRISK
          en:KSChangeDisp = DISPLAY_ASTERISK;
        state GOOD_UNLOCK
          (default) -> (junction 3490)
          (junction 3490) -> (junction 3491) [order 1] : [((ee_OwnerPassword[0] == pwd_Keyless[0])...
              |    && (ee_OwnerPassword[1] == pwd_Keyless[1])...
              |    && (ee_OwnerPassword[2] == pwd_Keyless[2])...
              |    && (ee_OwnerPassword[3] == pwd_Keyless[3])...
              |    && (ee_OwnerPassword[4] == pwd_Keyless[4]))...
              | || ((ee_MasterPassword[0] == pwd_Keyless[0])...
              |    && (ee_MasterPassword[1] == pwd_Keyless[1])...
              |    && (ee_MasterPassword[2] == pwd_Keyless[2])...
              |    && (ee_MasterPassword[3] == pwd_Keyless[3])...
              |    && (ee_MasterPassword[4] == pwd_Keyless[4]))]
          (junction 3490) -> (junction 3492) [order 2] : {
              | pwd_Position = 0;
              | pwd_Keyless = 0;
              | state_KSPassword = FAIL;
              | }
          (junction 3491) -> (junction 3493) : {
              | KSPWOwner = 1;
              | pwd_Position = 0;
              | pwd_Keyless = 0;
              | state_KSPassword = PASS;
              | }
          (junction 3493) -> (junction 3492)
        (default) -> DISPLAY_CODE
        DISPLAY_CODE -> KS_PASSWORD.CHANGE.ENTER_OWNER.DISPLAY_ASTRISK : [(pwd_Position != 0)]
        KS_PASSWORD.CHANGE.ENTER_OWNER.DISPLAY_ASTRISK -> KS_PASSWORD.CHANGE.ENTER_OWNER.GOOD_UNLOCK : [(pwd_Position == MAX_PWD_CHAR)]
      state OWNER_USER_SELECT
        state OWNER_USER_SELECT
          en:state_KSPassword = ENTER_OWNER1;
          en:DBEnterTime = 0;
          du:DBEnterTime++;
          state DISPLAY_OWNER
            en:PWD_NumberSelected = PW_OWNER;
            en:KSChangeDisp = DISPLAY_OWNER;
          state DISPLAY_DELAY
            state DISPLAY_DELAY_OWNER
            state DISPLAY_ENTER
              en:KSChangeDisp = DISPLAY_OWNER1;
              en:DBEnterTime = 0;
            (default) -> DISPLAY_DELAY_OWNER
            DISPLAY_DELAY_OWNER -> KS_PASSWORD.CHANGE.OWNER_USER_SELECT.OWNER_USER_SELECT.DISPLAY_DELAY.DISPLAY_ENTER : after(100,tick)
          state DISPLAY_USER1
            en:PWD_NumberSelected = PW_USER1;
            en:KSChangeDisp = DISPLAY_USER1;
          state DISPLAY_DELAY1
            state DISPLAY_DELAY_USER1
            state DISPLAY_ENTER
              en:KSChangeDisp = DISPLAY_OWNER1;
              en:DBEnterTime = 0;
            (default) -> DISPLAY_DELAY_USER1
            DISPLAY_DELAY_USER1 -> KS_PASSWORD.CHANGE.OWNER_USER_SELECT.OWNER_USER_SELECT.DISPLAY_DELAY1.DISPLAY_ENTER : after(100,tick)
          state DISPLAY_USER2
            en:PWD_NumberSelected = PW_USER2;
            en:KSChangeDisp = DISPLAY_USER2;
          state DISPLAY_DELAY2
            state DISPLAY_DELAY_USER2
            state DISPLAY_ENTER
              en:KSChangeDisp = DISPLAY_OWNER1;
              en:DBEnterTime = 0;
            (default) -> DISPLAY_DELAY_USER2
            DISPLAY_DELAY_USER2 -> KS_PASSWORD.CHANGE.OWNER_USER_SELECT.OWNER_USER_SELECT.DISPLAY_DELAY2.DISPLAY_ENTER : after(100,tick)
          (default) -> DISPLAY_OWNER
          DISPLAY_DELAY2 -> DISPLAY_OWNER : [((can_Keypad[2] & KEYPAD_UNLOCK_MASK) == KEYPAD_UNLOCK_PRESSED) &&...
              |  (state_KeylessPanel == NORMAL)]
          DISPLAY_OWNER -> DISPLAY_DELAY : [((can_Keypad[2] & KEYPAD_UNLOCK_MASK) != KEYPAD_UNLOCK_PRESSED)]
          DISPLAY_DELAY -> DISPLAY_USER1 : [((can_Keypad[2] & KEYPAD_UNLOCK_MASK) == KEYPAD_UNLOCK_PRESSED) &&...
              |  (state_KeylessPanel == NORMAL)]
          DISPLAY_USER1 -> DISPLAY_DELAY1 : [((can_Keypad[2] & KEYPAD_UNLOCK_MASK) != KEYPAD_UNLOCK_PRESSED)]
          DISPLAY_DELAY1 -> DISPLAY_USER2 : [((can_Keypad[2] & KEYPAD_UNLOCK_MASK) == KEYPAD_UNLOCK_PRESSED) &&...
              |  (state_KeylessPanel == NORMAL)]
          DISPLAY_USER2 -> DISPLAY_DELAY2 : [((can_Keypad[2] & KEYPAD_UNLOCK_MASK) != KEYPAD_UNLOCK_PRESSED)]
        state DISPLAY_ASTRISK
          en:KSChangeDisp = DISPLAY_ASTERISK;
          en:DBEnterTime = 0;
          du:DBEnterTime++;
        (default) -> KS_PASSWORD.CHANGE.OWNER_USER_SELECT.OWNER_USER_SELECT
        KS_PASSWORD.CHANGE.OWNER_USER_SELECT.OWNER_USER_SELECT -> KS_PASSWORD.CHANGE.OWNER_USER_SELECT.DISPLAY_ASTRISK : [(pwd_Position != 0)]
      state VERIFY_FIRST_ENTRY
        (default) -> (junction 3514)
        (junction 3514) -> (junction 3515) [order 1] : [!((pwd_Keyless[0] == ASCII_0) &&...
            |    (pwd_Keyless[1] == ASCII_0) &&...
            |    (pwd_Keyless[2] == ASCII_0) &&...
            |    (pwd_Keyless[3] == ASCII_0) &&...
            |    (pwd_Keyless[4] == ASCII_0))]
        (junction 3514) -> (junction 3516) [order 2] : {
            | state_KSPassword = FAIL;
            | }
        (junction 3515) -> (junction 3517) : {
            | pwd_New = pwd_Keyless;
            | pwd_Keyless = 0;
            | pwd_Position = 0;
            | state_KSPassword = PASS;
            | }
        (junction 3517) -> (junction 3516)
      state WAIT_NEW
        state DISPLAY_ASTRISK1
          en:state_KSPassword = ENTER_OWNER2;
          en:KSChangeDisp = DISPLAY_OWNER2;
        state DISPLAY_ASTRISK
          en:KSChangeDisp = DISPLAY_ASTERISK;
        (default) -> DISPLAY_ASTRISK1
        DISPLAY_ASTRISK1 -> KS_PASSWORD.CHANGE.WAIT_NEW.DISPLAY_ASTRISK : [(pwd_Position != 0)]
      state VERIFY_SECOND_ENTRY
        (default) -> (junction 3525)
        (junction 3525) -> (junction 3526) [order 1] : [(pwd_New[0] == pwd_Keyless[0])...
            |    && (pwd_New[1] == pwd_Keyless[1])...
            |    && (pwd_New[2] == pwd_Keyless[2])...
            |    && (pwd_New[3] == pwd_Keyless[3])...
            |    && (pwd_New[4] == pwd_Keyless[4])]
        (junction 3525) -> (junction 3527) [order 2] : {
            | state_KSPassword = FAIL;
            | }
        (junction 3526) -> (junction 3528) : {
            | state_KSPassword = PASS;
            | }
        (junction 3528) -> (junction 3527)
      state STORE
        en:state_KSPassword = NEW;
        en:KSChangeDisp = DISPLAY_SAVED;
      state GOOD_NEW
      state WAIT_NEW2
        en:state_KSPassword = 0;
      (default) -> ENTER_OWNER
      ENTER_OWNER -> WAIT_NEW1 [order 1] : after(CD_KEYPAD_ENTER_TIME,tick)
      ENTER_OWNER -> KS_PASSWORD.CHANGE.OWNER_USER_SELECT [order 2] : [(state_KSPassword == PASS)]
      KS_PASSWORD.CHANGE.OWNER_USER_SELECT -> WAIT_NEW1 [order 1] : [(DBEnterTime > CD_KEYPAD_ENTER_TIME)]
      KS_PASSWORD.CHANGE.OWNER_USER_SELECT -> VERIFY_FIRST_ENTRY [order 2] : [(pwd_Position == MAX_PWD_CHAR)]
      VERIFY_FIRST_ENTRY -> WAIT_NEW : [(state_KSPassword == PASS)]
      WAIT_NEW -> WAIT_NEW1 [order 1] : after(CD_KEYPAD_ENTER_TIME,tick)
      WAIT_NEW -> VERIFY_SECOND_ENTRY [order 2] : [(pwd_Position == MAX_PWD_CHAR)]
      VERIFY_SECOND_ENTRY -> STORE : [(state_KSPassword == PASS)]
      STORE -> GOOD_NEW : after(100,tick)
      GOOD_NEW -> WAIT_NEW2 [order 3] : [(PWD_NumberSelected == PW_OWNER) &&...
          |  (ee_OwnerPassword[0] == pwd_Keyless[0]) &&...
          |  (ee_OwnerPassword[1] == pwd_Keyless[1]) &&...
          |  (ee_OwnerPassword[2] == pwd_Keyless[2]) &&...
          |  (ee_OwnerPassword[3] == pwd_Keyless[3]) &&...
          |  (ee_OwnerPassword[4] == pwd_Keyless[4])]
      GOOD_NEW -> WAIT_NEW2 [order 1] : [(PWD_NumberSelected == PW_USER1) &&...
          |  (ee_User1Password[0] == pwd_Keyless[0]) &&...
          |  (ee_User1Password[1] == pwd_Keyless[1]) &&...
          |  (ee_User1Password[2] == pwd_Keyless[2]) &&...
          |  (ee_User1Password[3] == pwd_Keyless[3]) &&...
          |  (ee_User1Password[4] == pwd_Keyless[4])]
      GOOD_NEW -> WAIT_NEW2 [order 2] : [(PWD_NumberSelected == PW_USER2) &&...
          |  (ee_User2Password[0] == pwd_Keyless[0]) &&...
          |  (ee_User2Password[1] == pwd_Keyless[1]) &&...
          |  (ee_User2Password[2] == pwd_Keyless[2]) &&...
          |  (ee_User2Password[3] == pwd_Keyless[3]) &&...
          |  (ee_User2Password[4] == pwd_Keyless[4])]
    state NOTHING
      en:state_KSPassword = 0;
      en:pwd_Position = 0;
      en:pwd_Keyless = 0;
    state WAIT_UNLOCK_CHART
      en:state_KSPassword = ENTER_PWD;
    state WAIT_UNLOCK
    state FAIL
      en:state_KSPassword = FAIL;
    state GOOD_UNLOCK
      (default) -> (junction 3534)
      (junction 3534) -> (junction 3535) [order 1] : [((ee_OwnerPassword[0] == pwd_Keyless[0])...
          |    && (ee_OwnerPassword[1] == pwd_Keyless[1])...
          |    && (ee_OwnerPassword[2] == pwd_Keyless[2])...
          |    && (ee_OwnerPassword[3] == pwd_Keyless[3])...
          |    && (ee_OwnerPassword[4] == pwd_Keyless[4]))...
          | || ((ee_MasterPassword[0] == pwd_Keyless[0])...
          |    && (ee_MasterPassword[1] == pwd_Keyless[1])...
          |    && (ee_MasterPassword[2] == pwd_Keyless[2])...
          |    && (ee_MasterPassword[3] == pwd_Keyless[3])...
          |    && (ee_MasterPassword[4] == pwd_Keyless[4]))]
      (junction 3534) -> (junction 3536) [order 2]
      (junction 3535) -> (junction 3537) : {
          | KSPWOwner = 1;
          | }
      (junction 3536) -> (junction 3537) [order 1] : [((state_KSLock == KS_UNLOCK) ||...
          |   (state_KSLock == KS_LOCK)) &&...
          | (((ee_User1Password[0] == pwd_Keyless[0])...
          |    && (ee_User1Password[1] == pwd_Keyless[1])...
          |    && (ee_User1Password[2] == pwd_Keyless[2])...
          |    && (ee_User1Password[3] == pwd_Keyless[3])...
          |    && (ee_User1Password[4] == pwd_Keyless[4]))...
          | || ((ee_User2Password[0] == pwd_Keyless[0])...
          |    && (ee_User2Password[1] == pwd_Keyless[1])...
          |    && (ee_User2Password[2] == pwd_Keyless[2])...
          |    && (ee_User2Password[3] == pwd_Keyless[3])...
          |    && (ee_User2Password[4] == pwd_Keyless[4])))]
      (junction 3536) -> (junction 3538) [order 2] : {
          | state_KSPassword = FAIL;
          | }
      (junction 3537) -> (junction 3539) : {
          | state_KSPassword = PASS;
          | }
      (junction 3539) -> (junction 3538)
    (default) -> KS_PASSWORD.NOTHING
    KS_PASSWORD.NOTHING -> KS_PASSWORD.CHANGE [order 1] : [(state_KSLock == KS_CHANGE)]
    (junction 3461) -> KS_PASSWORD.NOTHING
    KS_PASSWORD.NOTHING -> WAIT_UNLOCK_CHART [order 2] : [(state_KSLock != KS_NOTHING)]
    KS_PASSWORD.CHANGE -> (junction 3461) [order 2] : [(state_KSPassword == 0)]
    KS_PASSWORD.CHANGE -> FAIL [order 1] : [(state_KSPassword == FAIL)]
    WAIT_UNLOCK_CHART -> WAIT_UNLOCK : [(pwd_Position != 0)]
    FAIL -> (junction 3461) : after(100,tick)[(reset_PwdState)]
    KS_PASSWORD.GOOD_UNLOCK -> (junction 3461) [order 1] : after(50,tick)[(state_KSLock == KS_NOTHING)...
        | && (state_KSPassword != FAIL)]
        | {
        | pwd_Position = 0;
        | pwd_Keyless = 0;
        | }
    WAIT_UNLOCK -> FAIL [order 1] : after(CD_KEYPAD_ENTER_TIME, tick)
    WAIT_UNLOCK -> KS_PASSWORD.GOOD_UNLOCK [order 2] : [(pwd_Position == MAX_PWD_CHAR)]
    KS_PASSWORD.GOOD_UNLOCK -> FAIL [order 2] : [(state_KSPassword == FAIL)]
  state KS_LOCK [parallel]
    settings: executionOrder=3
    state Init
      en:state_KSLock = KS_NOTHING;
    state NOTHING
      en:state_KSLock = KS_NOTHING;
    state MACHINE_UNLOCK
      en:state_KSLock = KS_UNLOCK;
    state MACHINE_LOCK
      en:state_KSLock = KS_LOCK;
    state LOCK
      en:state_KSLock = KS_LOCK_MACHINE;
    state LOCK_DB
    state UNLOCK
      en:state_KSLock = KS_UNLOCK_MACHINE;
    state UNLOCK_DB
    state BUTTON_CHANGE_DB
      en:debounceCount = 0;
      du:debounceCount++;
    state CHANGE
      en:state_KSLock = KS_CHANGE;
    (default) -> Init
    Init -> KS_LOCK.NOTHING : [flag_InitEERead]
    MACHINE_UNLOCK -> KS_LOCK.NOTHING : [(state_MachineLock == UNLOCK)...
        | || (ee_PasswordLock == INACTIVE)]
    MACHINE_LOCK -> MACHINE_UNLOCK [order 2] : [(state_KSPassword == PASS)]
    KS_LOCK.NOTHING -> MACHINE_LOCK [order 1] : [(state_MachineLock == LOCKED)...
        | && (ee_PasswordLock == ACTIVE)]
    MACHINE_LOCK -> KS_LOCK.NOTHING [order 1] : [(ee_PasswordLock == INACTIVE)]
    LOCK -> MACHINE_LOCK [order 1] : [(ee_PasswordLock == LOCKED)]
    KS_LOCK.NOTHING -> LOCK_DB [order 4] : [((can_Keypad[2] & KEYPAD_LOCK_MASK) == KEYPAD_LOCK_PRESSED)...
        | && ((can_Keypad[2] & KEYPAD_UNLOCK_MASK) == 0)...
        | && (ee_PasswordLock == UNLOCK)...
        | && (state_KeylessPanel == NORMAL)...
        | && ((state_Engine == ENGINE_NOT_RUNNING) || (state_Engine == ENGINE_INITIALIZE))]
    LOCK_DB -> LOCK [order 2] : [after(50,tick)]
    LOCK_DB -> KS_LOCK.NOTHING [order 1] : [((can_Keypad[2] & KEYPAD_UNLOCK_MASK) == KEYPAD_UNLOCK_PRESSED)]
    LOCK -> KS_LOCK.NOTHING [order 2] : [(state_KSPassword == FAIL)]
    KS_LOCK.NOTHING -> UNLOCK_DB [order 2] : [((can_Keypad[2] & KEYPAD_UNLOCK_MASK) == KEYPAD_UNLOCK_PRESSED)...
        | && ((can_Keypad[2] & KEYPAD_LOCK_MASK) == 0)...
        | && (ee_PasswordLock == LOCKED)...
        | && (state_KeylessPanel == NORMAL)...
        | && ((state_Engine == ENGINE_NOT_RUNNING) || (state_Engine == ENGINE_INITIALIZE))]
    UNLOCK_DB -> UNLOCK [order 1] : [after(50,tick)]
    UNLOCK_DB -> KS_LOCK.NOTHING [order 2] : [((can_Keypad[2] & KEYPAD_LOCK_MASK) == KEYPAD_LOCK_PRESSED)]
    UNLOCK -> KS_LOCK.NOTHING : [(ee_PasswordLock == UNLOCK)...
        | || (state_KSPassword == FAIL)]
    KS_LOCK.NOTHING -> BUTTON_CHANGE_DB [order 3] : [((can_Keypad[2] & KEYPAD_LOCK_MASK) == KEYPAD_LOCK_PRESSED)...
        | && ((can_Keypad[2] & KEYPAD_UNLOCK_MASK) == KEYPAD_UNLOCK_PRESSED)...
        | && (state_KeylessPanel == NORMAL)...
        | && ((state_Engine == ENGINE_NOT_RUNNING) || (state_Engine == ENGINE_INITIALIZE))]
    BUTTON_CHANGE_DB -> KS_LOCK.NOTHING [order 1] : [((can_Keypad[2] & KEYPAD_LOCK_MASK) == 0)...
        | || ((can_Keypad[2] & KEYPAD_UNLOCK_MASK) == 0)...
        | || (state_KeylessPanel != NORMAL)]
    BUTTON_CHANGE_DB -> KS_LOCK.CHANGE [order 2] : [(debounceCount >= 200)]
    KS_LOCK.CHANGE -> KS_LOCK.NOTHING : [(state_KSPassword == 0)...
        | || (state_KSPassword == FAIL)]
  state KS_DISPLAY [parallel]
    settings: executionOrder=4
    state CODE_ERROR2
      en:KSS_Display = DISPLAY_ERROR;
      state NOORMAL
        en:KSS_Display = INACTIVE;
      state PASSWORD
        state CODE
          en:KSS_Display = DISPLAY_CODE;
        state ASTERISK
          en:KSS_Display = DISPLAY_ASTERISK;
        (default) -> CODE
        CODE -> ASTERISK : [(pwd_Position != 0)]
      state CHANGE
        du: KSS_Display = KSChangeDisp;
      state CODE_ERROR
        en:KSS_Display = DISPLAY_ERROR;
      (default) -> NOORMAL
      PASSWORD -> NOORMAL [order 2] : after(50, tick)[(!(state_MachineLock) &&...
          |          ((state_KSLock == KS_UNLOCK) ||...
          |           (state_KSLock == KS_NOTHING)) &&...
          |           (state_KSPassword != FAIL))]
      CODE_ERROR -> NOORMAL : after(100,tick)[(state_KSPassword != FAIL)]
      KS_DISPLAY.CODE_ERROR2.CHANGE -> NOORMAL [order 2] : [(state_KSLock == KS_NOTHING)]
      NOORMAL -> PASSWORD [order 2] : [((ee_Keypad != INACTIVE) &&...
          |   (((state_MachineLock) && (state_KSLock == KS_LOCK)) ||...
          |  (state_KSLock == KS_UNLOCK_MACHINE) ||...
          |  (state_KSLock == KS_LOCK_MACHINE)))]
      NOORMAL -> KS_DISPLAY.CODE_ERROR2.CHANGE [order 1] : [(state_KSLock == KS_CHANGE)]
      PASSWORD -> CODE_ERROR [order 1] : [(state_KSPassword == FAIL)]
      KS_DISPLAY.CODE_ERROR2.CHANGE -> CODE_ERROR [order 1] : [(state_KSPassword == FAIL)]
    state CODE_ERROR1
      en:KSS_Display = DISPLAY_DELUXE_ERROR;
    (default) -> CODE_ERROR2
    CODE_ERROR2 -> CODE_ERROR1 : [state_KeylessPanel == NO_COMMUNICATION]
  data: ee_Keypad data_store_memory double; can_Keypad input uint8 size=3; state_KeylessPanel input uint8; state_KSLock output uint8; ee_PasswordLock data_store_memory uint8; debounceCount local uint16; KEYPAD_LOCK_MASK parameter uint8; KEYPAD_LOCK_PRESSED parameter uint8; KEYPAD_UNLOCK_MASK parameter uint8; KEYPAD_UNLOCK_PRESSED parameter uint8; NORMAL parameter uint8; PASS parameter uint8; FAIL parameter uint8; state_KSPassword output uint8; KS_LOCK parameter uint8; KS_UNLOCK parameter uint8; KS_CHANGE parameter uint8; KS_NOTHING parameter uint8; NEW parameter uint8; LOCKED parameter uint8; UNLOCK parameter uint8; pwd_Position output uint8; pwd_Keyless output uint8 size=5; ASCII_1 parameter uint8; ASCII_2 parameter uint8; ASCII_3 parameter uint8; ASCII_4 parameter uint8; ASCII_5 parameter uint8; ASCII_6 parameter uint8; ASCII_7 parameter uint8; ASCII_8 parameter uint8; ASCII_9 parameter uint8; ASCII_0 parameter uint8; KEYPAD_PRESSED_1 parameter uint8; KEYPAD_MASK_1 parameter uint8; KEYPAD_MASK_2 parameter uint8; KEYPAD_PRESSED_2 parameter uint8; KEYPAD_MASK_3 parameter uint8; KEYPAD_PRESSED_3 parameter uint8; KEYPAD_MASK_4 parameter uint8; KEYPAD_PRESSED_4 parameter uint8; KEYPAD_MASK_5 parameter uint8; KEYPAD_PRESSED_5 parameter uint8; KEYPAD_MASK_6 parameter uint8; KEYPAD_PRESSED_6 parameter uint8; KEYPAD_MASK_7 parameter uint8; KEYPAD_PRESSED_7 parameter uint8; KEYPAD_MASK_8 parameter uint8; KEYPAD_PRESSED_8 parameter uint8; KEYPAD_MASK_9 parameter uint8; KEYPAD_PRESSED_9 parameter uint8; KEYPAD_MASK_0 parameter uint8; KEYPAD_PRESSED_0 parameter uint8; MAX_PWD_CHAR parameter uint8; ee_OwnerPassword input uint8 size=5; ee_MasterPassword input uint8 size=5; ee_User1Password input uint8 size=5; ee_User2Password input uint8 size=5; reset_PwdState local uint8; KS_LOCK_MACHINE parameter uint8; KS_UNLOCK_MACHINE parameter uint8; ENTER_OWNER1 parameter uint8; ACTIVE parameter uint8; INACTIVE parameter uint8; CD_KEYPAD_ENTER_TIME parameter uint16; pwd_New local uint8 size=5; ENTER_OWNER2 parameter uint8; ENTER_PWD parameter uint8; KSS_Display output uint8; state_MachineLock input uint8; DISPLAY_OWNER2 parameter uint8; DISPLAY_OWNER1 parameter uint8; DISPLAY_ERROR parameter uint8; DISPLAY_CODE parameter uint8; DISPLAY_ASTERISK parameter uint8; NO_COMMUNICATION parameter uint8; DISPLAY_DELUXE_ERROR parameter uint8; DISPLAY_SAVED parameter uint8; KSChangeDisp local uint8; PW_OWNER parameter uint8; PW_USER1 parameter uint8; PW_USER2 parameter uint8; DISPLAY_USER1 parameter uint8; DISPLAY_USER2 parameter uint8; PWD_NumberSelected output uint16; KSPWOwner local boolean; DBEnterTime local uint16; state_Engine input uint8; DISPLAY_OWNER parameter uint8; ENGINE_INITIALIZE parameter uint8; ENGINE_NOT_RUNNING parameter uint8; flag_InitEERead input boolean
